simulink model "PlanarModelandControl"  (R2026a)
settings: Solver=FixedStepDiscrete  StopTime=StopTime  FixedStep=Ts  MaxStep=0  RelTol=1e-5  AbsTol=1e-3
PostLoadFcn:
  | Controles_Ativos = 1;
  | Vx_Ref = 0;
  | Vx_init = 0;
  | t_default = [0; 10000];
  | Brake_Cmd = [t_default, [0; 0]];
  | Accel_Cmd = [t_default, [0; 0]];
  | Steer_Cmd = [t_default, [0; 0]];
  | Flag_CruiseControl = 0;
  | Ts = 0.001;
  | load('TC_LUT.mat')
InitFcn:
  | % Carrega parâmetros gerais (Massa, Ganhos PID, Geometria)
  | run('Parameters.m');
  | 
  | % Carrega as Tabelas (Lookup Tables)
  | run('curva_steering.m');
  | run('curva_tq_motor.m');
  | load('TC_LUT.mat')
model workspace (MAT data in the model file):
  A = 1.22
  Ack = 1
  Cd = 1.1
  Cl = -2
  Cs = [19075.58 25992.8 31792.64 35898.02 39044.37]
  Fz0x = -811.9083
  Fz0y = -210.9467
  Fz_cs = [222.41 444.82 667.23 889.64 1112.05]
  IAY = 0
  IAx = 0
  IAy = 0
  Im = 0.00033
  It = 0.0094
  Iw = 0.1578
  Iw_ = 0.7
  Iz_car = 180
  K_50_understeer = 0.005184
  K_Stability = 1
  K_oversteer = -0.001
  Kappa_F = 0
  Kappa_R = 0
  Km_FM = 110000
  Km_Fm = 110000
  Km_RM = 120000
  Km_Rm = 120000
  KphiARB_F = 0
  KphiARB_R = 11000
  KphiF = 17054.5
  KphiR = 40034.8
  Kphim_F = 137500
  Kphim_R = 48600
  L = 1.53
  M = 300
  Mgt = 11.72
  P = 68.9476
  P0 = 55.4852
  R = 0.2032
  Rcurva = 20
  Re = 0.2
  SR = 3
  TV_On = 1
  Ts = 0.001
  W = 2940
  Wf = 308.034
  Wr = 339.426
  Ws = 2099.34
  YLL = 1.11022302463e-16
  cx1 = 1.3668
  cy1 = 1.839
  dpi = 0.242630467224
  dx1 = 2.1568
  dx2 = -0.065814
  dx3 = 0.0011254
  dy1 = 2.453
  dy2 = -0.11197
  dy3 = 10.2899
  ex1 = -0.19815
  ex2 = -0.62529
  ex3 = 1.3396
  ex4 = 0.0029464
  ey1 = 0.96085
  ey2 = -0.28662
  ey3 = 0.14389
  ey4 = 4.9274
  ey5 = -70.7165
  g = 9.81
  hcg = 0.252
  hcgs = 0.202
  hrc_f = 0.056
  hrc_r = 0.084
  hs_f = 0.222
  hs_r = 0.222
  hx1 = -0.003859
  hx2 = -0.000374
  hy1 = -0.000403
  hy2 = -0.000261
  kx1 = -36.9039
  kx2 = 22.3857
  kx3 = 0.61844
  ky1 = -83.2526
  ky2 = -5.565
  ky3 = 0.88491
  ky4 = 2.9994
  ky5 = -99.9393
  ky6 = -3.4705
  ky7 = -0.91452
  lf = 0.8415
  lr = 0.6885
  mass_FL = 67.5
  mass_FR = 67.5
  mass_RL = 82.5
  mass_RR = 82.5
  mi = 2
  ms = 240
  pty1 = 0.005744
  pty2 = 19.1334
  px1 = -0.039964
  px2 = -0.15015
  px3 = -0.32528
  px4 = -0.063273
  py1 = 0.38578
  py2 = 1.2048
  py3 = -0.14544
  py4 = 0.092886
  py5 = -0.82901
  r = 9
  rhoar = 1.22
  s_f = 1
  s_r = 0.9
  tf = 1.25
  tr = 1.25
  vx1 = -0.11922
  vx2 = 0.002205
  vy1 = 0.032907
  vy2 = 0.018068
  vy3 = -1.0429
  vy4 = 0.53712
  x_fl = 0.8415
  x_fr = 0.8415
  x_rl = 0.6885
  x_rr = 0.6885
  xs_f = 0.826
  xs_r = 0.704
  y_fl = 0.6
  y_fr = 0.6
  y_rl = 0.59
  y_rr = 0.59
values from neighbouring files (symbols this model uses):
  GG_mode = 0    [autocross.m]    (also in teste.mat)
  Gr = 11.72    [Parameters.m]    (also in teste.mat)
  K_variavel = [-0.001, 0, 0.0008, 0.0012, 0.0015]    [Parameters.m]    (also in teste.mat = [-0.001 0 0.0008 0.0012 0.0015])
  Ki_data = [283117.9493, 114831.615, 51596.0519, 30556.5344, 17525.221, 11773.8301, 10567.5001]*0.008    [Parameters.m]    (also in teste.mat = [2264.9435944 918.65292 412.7684152 244.4522752 140.201768 9)
  Kp_data = [2877.5702, 2303.9999, 1972.0835, 1556.8775, 1258.2451, 1022.7085, 982.7861, ]*5    [Parameters.m]    (also in teste.mat = [14387.851 11519.9995 9860.4175 7784.3875 6291.2255 5113.542)
  Mz_maxF = [0 66.3 132.6 198.9 265.2 330.23 396.52 462.83 529.112 595.42 661.73 728.03 794.33 860.62 926.92 993.23 1058.25 1124.55 1190.85 1257.15 1323.45 1389.75 1456.05 1522.35 1588.65 1654.95]    [Parameters.m]    (also in teste.mat = [0 66.3 132.6 198.9 265.2 330.23 396.52 462.83 529.112 595.4)
  Mz_minF = [0 -66.3 -132.6 -198.9 -265.2 -330.23 -396.52 -462.83 -529.112 -595.42 -661.73 -728.03 -794.33 -860.62 -926.92 -993.23 -1058.25 -1124.55 -1190.85 -1257.15 -1323.45 -1389.75 -1456.05 -1522.35 -1588.65 -1654.95]    [Parameters.m]    (also in teste.mat = [0 -66.3 -132.6 -198.9 -265.2 -330.23 -396.52 -462.83 -529.1)
  pedal = [0 4 8 12 16 20 24 28 32 36 40 44 48 52 56 60 64 68 72 76 80 84 88 92 96 100]    [Parameters.m]    (also in teste.mat = [0 4 8 12 16 20 24 28 32 36 40 44 ... (26 elements, 1x26)])
  rpm_355_novo = [0 10050 10100 10140 10170 10210 10250 10280 10320 10360         10390 10420 10460 10620 10770 10920 11050 11170 11280         11380 11470 11550 11625 11680 11730 11775 11790 11800]    [curva_tq_motor.m]    (also in teste.mat = [0 10050 10100 10140 10170 10210 10250 10280 10320 10360 103)
  Steering_inner = [-29.018 ... (first row only)    [curva_steering.m]    (also in teste.mat = [-29.018 -25.595 -22.357 -19.265 -16.292 -13.415 -10.619 -7.)
  Steering_outer = [-25.111 ... (first row only)    [curva_steering.m]    (also in teste.mat = [-25.111 -22.589 -20.082 -17.585 -15.094 -12.604 -10.11 -7.6)
  steering_vol = [-105 ... (first row only)    [curva_steering.m]    (also in teste.mat = [-105 -94.5 -84 -73.5 -63 -52.5 -42 -31.5 -21 -10.5 0 10.5 .)
  torque_355_novo = [16.2 16.2 16.0 15.8 15.6 15.4 15.2 15.0 14.8 14.6            14.4 14.2 14.0 13.0 12.0 11.0 10.0 9.0 8.0            7.0 6.0 5.0 4.0 3.0 2.0 1.0 0.5 0.0]    [curva_tq_motor.m]    (also in teste.mat = [16.2 16.2 16 15.8 15.6 15.4 15.2 15 14.8 14.6 14.4 14.2 ...)
  Vx = [0, 12.5, 23.6, 30, 35]    [Parameters.m]    (also in teste.mat = [0 12.5 23.6 30 35])
  Vx_axis = [5, 10, 15, 20, 25, 30, 35]    [Parameters.m]    (also in teste.mat = [5 10 15 20 25 30 35])
undefined symbols (not defined in the file or in its folder): DiscIntNeverNeededParam, magnitude
libraries: simulink x5, fuzblock x4, sl_discontinuities x3, simulink_extras x3, slpidlib x1
(part 1 of 4)
settings Model: SimulationMode=accelerator  SILPILModeSetting=simonly  SILPILSimulationModeTopModel=accelerator  PauseTimes=21.560  Creator=lucas  LastModifiedBy=lucaspz
settings SolverCC: ZeroCrossAlgorithm=Adaptive
settings DebuggingCC: SignalInfNanChecking=warning  IgnoredZcDiagnostic=none
settings SimscapeCC: SimscapeLogDecimation=1  SimscapeLogDataHistory=5000  SimscapeLogType=none  SimscapeCacheCompileArtifacts=�  SimscapeAutoSelectReusableComponents=�  SimscapeNominalValues="[{\"value\":\"1\",\"unit\":\"A\"},{\"value\":\"1\",\"unit\":\"bar\"},{\"value\":\"1\",\"unit\":\"cm^2\"},{\"value\":\"1\",\"unit\":\"cm^3/s\"},{\"value\":\"1\",\"unit\":\"kJ/kg\"},{\"value\":\"1\",\"unit\":\"kW\"},{\"value\":\"1\",\"unit\":\"l\"},{\"value\":\"1\",\"unit\":\"N\"},{\"value\":\"1\",\"unit\":\"N*m\"},{\"value\":\"1\",\"unit\":\"V\"}]"

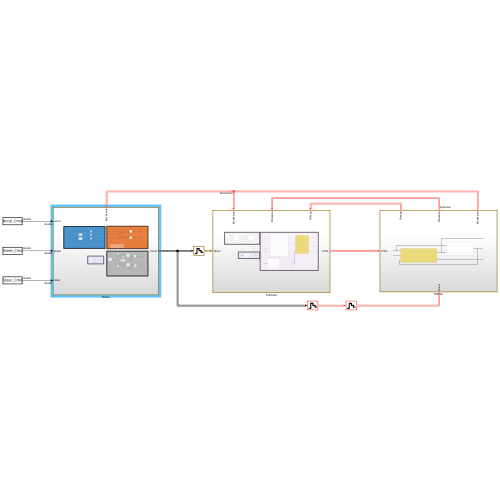
[thumbnail: Simulink's stored preview of the top-level diagram]
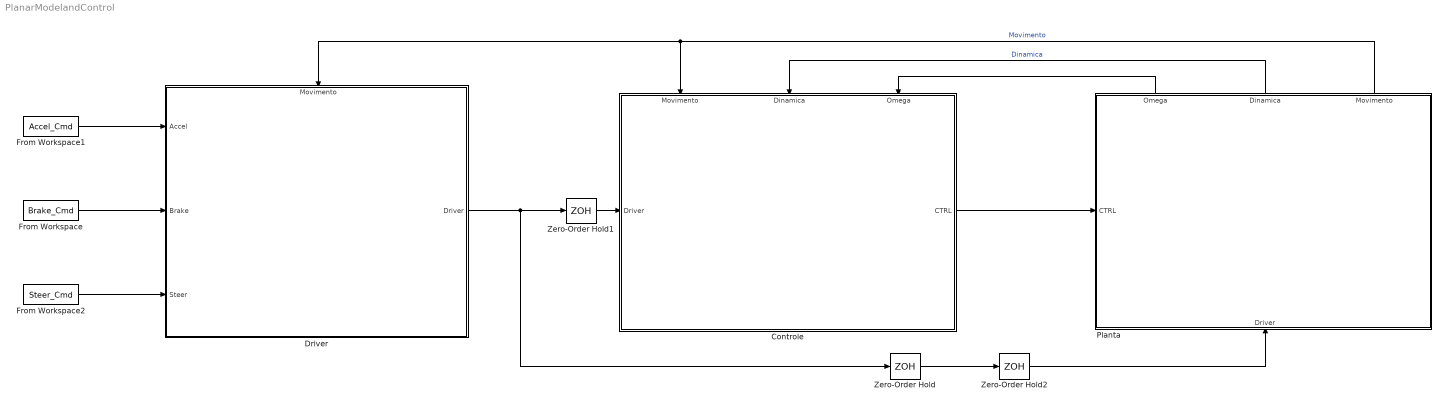
== system PlanarModelandControl ==
  Controle : Subsystem
      settings: PortSchema="{\"entries\":[{\"content\":{\"sides\":[{\"content\":{\"connectorIds\":[\"In1\"]},\"type\":\"ConnectorPlacement.EquallySpacedRectSide\",\"uuid\":\"56151e0a-bfa8-4f54-a24f-2d0820c0f72f\"},{\"content\":{\"connectorIds\":[\"Out1\"],\"side\":\"RIGHT\"},\"type\":\"ConnectorPlacement.EquallySpacedRectSide\",\"uuid\":\"009f5130-1eb0-405e-8bd9-87ce9896eab4\"},{\"content\":{\"connectorIds\":[\"In2\",\"In3\",\"In4\"],\"side\":\"TOP\"},\"type\":\"ConnectorPlacement.EquallySpacedRectSide\",\"uuid\":\"a6bcbcd6-40f0-4fd8-bd63-4ee066739afa\"},{\"content\":{\"side\":\"BOTTOM\"},\"type\":\"ConnectorPlacement.EquallySpacedRectSide\",\"uuid\":\"b3b13c4c-f99f-4d3e-8267-66104372a3a2\"}]},\"type\":\"ConnectorPlacement.EquallySpacedConnector\",\"uuid\":\"073272d2-a218-475e-9766-74f02117ed8f\"}],\"packageUris\":[\"http://schema.mathworks.com/mf0/Connector/R2020b\"],\"version\":\"1.0\"}"
  Driver : Subsystem
      settings: PortSchema="{\"entries\":[{\"content\":{\"sides\":[{\"content\":{\"connectorIds\":[\"In1\",\"In2\",\"In3\"]},\"type\":\"ConnectorPlacement.EquallySpacedRectSide\",\"uuid\":\"443768bc-c7a3-4466-aec1-096d5f7be67d\"},{\"content\":{\"connectorIds\":[\"Out1\"],\"side\":\"RIGHT\"},\"type\":\"ConnectorPlacement.EquallySpacedRectSide\",\"uuid\":\"da7fa586-0510-4892-a092-db6f1a27a2ae\"},{\"content\":{\"connectorIds\":[\"In4\"],\"side\":\"TOP\"},\"type\":\"ConnectorPlacement.EquallySpacedRectSide\",\"uuid\":\"2c1d5f3e-1036-4d42-9b19-272a34ee3261\"}]},\"type\":\"ConnectorPlacement.EquallySpacedConnector\",\"uuid\":\"e3719b6f-9fe7-4b22-84b4-1211ae849bc8\"}],\"packageUris\":[\"http://schema.mathworks.com/mf0/Connector/R2020b\"],\"version\":\"1.0\"}"
  From Workspace : FromWorkspace  VariableName=Brake_Cmd
  From Workspace1 : FromWorkspace  VariableName=Accel_Cmd
  From Workspace2 : FromWorkspace  VariableName=Steer_Cmd
  Planta : Subsystem
      settings: PortSchema="{\"entries\":[{\"content\":{\"sides\":[{\"content\":{\"connectorIds\":[\"In1\"]},\"type\":\"ConnectorPlacement.EquallySpacedRectSide\",\"uuid\":\"52b90d9a-1301-422a-8be6-7849c42227ef\"},{\"content\":{\"side\":\"RIGHT\"},\"type\":\"ConnectorPlacement.EquallySpacedRectSide\",\"uuid\":\"dc52c6c3-a926-4968-a1e6-05499ba453a3\"},{\"content\":{\"connectorIds\":[\"Out1\",\"Out2\",\"Out3\"],\"side\":\"TOP\"},\"type\":\"ConnectorPlacement.EquallySpacedRectSide\",\"uuid\":\"4b5251a8-57d3-4280-b8f2-cf87664bd53d\"},{\"content\":{\"connectorIds\":[\"In2\"],\"side\":\"BOTTOM\"},\"type\":\"ConnectorPlacement.EquallySpacedRectSide\",\"uuid\":\"b553b3b1-29bc-46a4-a447-2da3a1af9f5a\"}]},\"type\":\"ConnectorPlacement.EquallySpacedConnector\",\"uuid\":\"f03778a7-5380-42c5-afb8-9cbdce520324\"}],\"packageUris\":[\"http://schema.mathworks.com/mf0/Connector/R2020b\"],\"version\":\"1.0\"}"
  Zero-Order Hold : ZeroOrderHold  SampleTime=0.001
  Zero-Order Hold1 : ZeroOrderHold  SampleTime=0.01
  Zero-Order Hold2 : ZeroOrderHold  SampleTime=0.001
  wires:
  Driver/1(Driver) -> Zero-Order Hold/1, Zero-Order Hold1/1
  Zero-Order Hold/1 -> Zero-Order Hold2/1
  Controle/1(CTRL) -> Planta/1(CTRL)
  Planta/2(Dinamica) -> Controle/3(Dinamica)  "Dinamica"
  Planta/3(Movimento) -> Driver/4(Movimento), Controle/2(Movimento)  "Movimento"
  Planta/1(Omega) -> Controle/4(Omega)
  From Workspace1/1 -> Driver/1(Accel)
  From Workspace/1 -> Driver/2(Brake)
  From Workspace2/1 -> Driver/3(Steer)
  Zero-Order Hold1/1 -> Controle/1(Driver)
  Zero-Order Hold2/1 -> Planta/2(Driver)

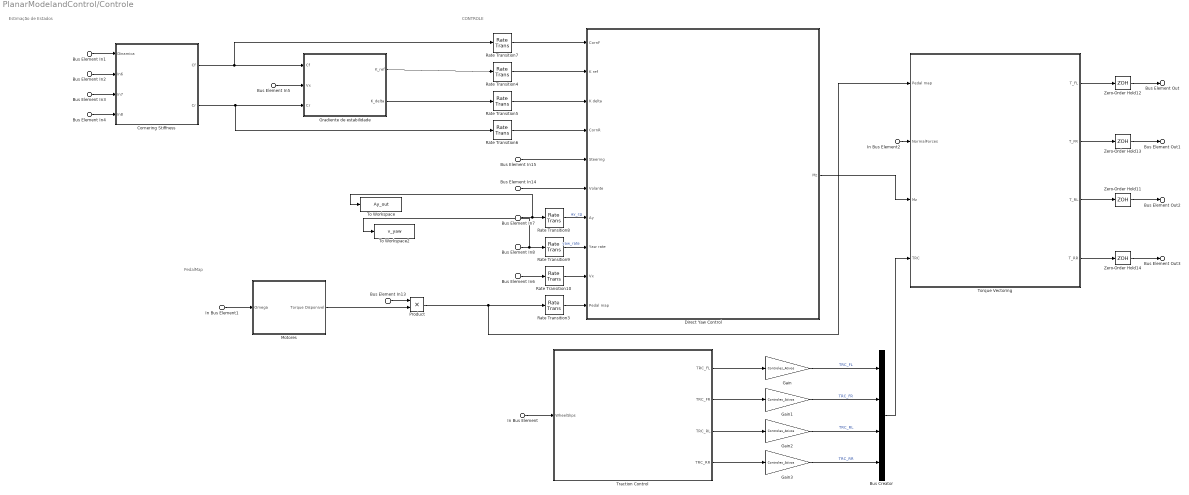
== system PlanarModelandControl/Controle ==
  note: PedalMap
  note: CONTROLE
  note: Estimação de Estados
  Bus Element In13 : Inport  PortName=Driver  Element=AccCmd
  Bus Element In14 : Inport  PortName=Driver  Element=volante
  Bus Element In15 : Inport  PortName=Driver  Element=steering_inner
  Bus Element In5 : Inport  Port=2  PortName=Movimento  Element=Vx_cg
  Bus Element In6 : Inport  Port=2  PortName=Movimento  Element=Vx_cg
  Bus Element In7 : Inport  Port=2  PortName=Movimento  Element=Ay_cg
  Bus Element In8 : Inport  Port=2  PortName=Movimento  Element=Yaw.v_yaw
  In Bus Element2 : Inport  Port=3  PortName=Dinamica  Element=NormalForces
  Bus Element In1 : Inport  Port=3  PortName=Dinamica  Element=NormalForces.Fz_FL
  Bus Element In2 : Inport  Port=3  PortName=Dinamica  Element=NormalForces.Fz_FR
  Bus Element In3 : Inport  Port=3  PortName=Dinamica  Element=NormalForces.Fz_RL
  Bus Element In4 : Inport  Port=3  PortName=Dinamica  Element=NormalForces.Fz_RR
  In Bus Element : Inport  Port=3  PortName=Dinamica  Element=WheelSlips
  In Bus Element1 : Inport  Port=4  PortName=Omega
  Bus Creator : BusCreator  Inputs=4
  Cornering Stiffness : Subsystem
  Direct Yaw Control : Subsystem
  Gain{,1..3} (4 alike) : Gain  Gain=Controles_Ativos
  Gradiente de estabilidade : Subsystem
  Motores : Subsystem
  Product : Product
  Rate Transition{3..10} (8 alike) : RateTransition  OutPortSampleTime=0.01
  To Workspace : ToWorkspace  VariableName=Ay_out  SaveFormat=Structure With Time  SampleTime=-1
  To Workspace2 : ToWorkspace  VariableName=v_yaw  SaveFormat=Structure With Time  SampleTime=-1
  Torque Vectoring : Subsystem  TreatAsAtomicUnit=on
  Traction Control : Subsystem
  Zero-Order Hold{11..14} (4 alike) : ZeroOrderHold  SampleTime=0.001
  Bus Element Out : Outport  VectorParamsAs1DForOutWhenUnconnected=off  PortName=CTRL  Element=T_FL
  Bus Element Out1 : Outport  VectorParamsAs1DForOutWhenUnconnected=off  PortName=CTRL  Element=T_FR
  Bus Element Out2 : Outport  VectorParamsAs1DForOutWhenUnconnected=off  PortName=CTRL  Element=T_RL
  Bus Element Out3 : Outport  VectorParamsAs1DForOutWhenUnconnected=off  PortName=CTRL  Element=T_RR
  wires:
  Bus Element In13/1 -> Product/1
  Bus Element In3/1 -> Cornering Stiffness/3(In7)
  Bus Element In1/1 -> Cornering Stiffness/1(Dinamica)
  Bus Element In4/1 -> Cornering Stiffness/4(In8)
  Bus Element In2/1 -> Cornering Stiffness/2(In6)
  Bus Element In14/1 -> Direct Yaw Control/6(Volante)
  Bus Element In7/1 -> To Workspace/1, Rate Transition8/1
  Bus Element In6/1 -> Rate Transition10/1
  Bus Element In15/1 -> Direct Yaw Control/5(Steering)
  Cornering Stiffness/1(Cf) -> Rate Transition7/1, Gradiente de estabilidade/1(Cf)
  Gradiente de estabilidade/2(K_delta) -> Rate Transition5/1
  Gradiente de estabilidade/1(K_ref) -> Rate Transition4/1
  Cornering Stiffness/2(Cr) -> Gradiente de estabilidade/3(Cr), Rate Transition6/1
  Torque Vectoring/1(T_FL) -> Zero-Order Hold12/1
  Torque Vectoring/2(T_FR) -> Zero-Order Hold13/1
  Torque Vectoring/4(T_RR) -> Zero-Order Hold14/1
  Bus Element In5/1 -> Gradiente de estabilidade/2(Vx)
  Motores/1(Torque Disponivel) -> Product/2
  Zero-Order Hold13/1 -> Bus Element Out1/1
  Zero-Order Hold11/1 -> Bus Element Out2/1
  Zero-Order Hold14/1 -> Bus Element Out3/1
  Gain/1 -> Bus Creator/1  "TRC_FL"
  Gain1/1 -> Bus Creator/2  "TRC_FR"
  Rate Transition3/1 -> Direct Yaw Control/10(Pedal map)
  Rate Transition4/1 -> Direct Yaw Control/2(K ref)
  Rate Transition5/1 -> Direct Yaw Control/3(K delta)
  Rate Transition6/1 -> Direct Yaw Control/4(CornR)
  Rate Transition7/1 -> Direct Yaw Control/1(CornF)
  Rate Transition8/1 -> Direct Yaw Control/7(Ay)  "ay_cg"
  Rate Transition9/1 -> Direct Yaw Control/8(Yaw rate)  "yaw_rate"
  Rate Transition10/1 -> Direct Yaw Control/9(Vx)
  Gain2/1 -> Bus Creator/3  "TRC_RL"
  Gain3/1 -> Bus Creator/4  "TRC_RR"
  Bus Element In8/1 -> To Workspace2/1, Rate Transition9/1
  Torque Vectoring/3(T_RL) -> Zero-Order Hold11/1
  Zero-Order Hold12/1 -> Bus Element Out/1
  Direct Yaw Control/1(Mz) -> Torque Vectoring/3(Mz)
  Traction Control/1(TRC_FL) -> Gain/1
  Traction Control/2(TRC_FR) -> Gain1/1
  Traction Control/3(TRC_RL) -> Gain2/1
  Traction Control/4(TRC_RR) -> Gain3/1
  Bus Creator/1 -> Torque Vectoring/4(TRC)
  In Bus Element/1 -> Traction Control/1(WheelSlips)
  In Bus Element1/1 -> Motores/1(Omega)
  In Bus Element2/1 -> Torque Vectoring/2(NormalForces)
  Product/1 -> Torque Vectoring/1(Pedal map), Rate Transition3/1

== system PlanarModelandControl/Driver ==
  note: positivo para esquerda --> left = inner e right = outer
  note: Curva de velocidade
  note: Indicador Brake/Acc
  note: Curva de Steering
  note: Indicador inner/outer
  note: negativo para direita --> left = outer e right = inner
  Bus Element In1 : Inport  PortName=Accel
  Bus Element In2 : Inport  Port=2  PortName=Brake
  Bus Element In3 : Inport  Port=3  PortName=Steer
  Bus Element In : Inport  Port=4  PortName=Movimento  Element=Vx_cg
  1-D Lookup Table2 : Lookup_n-D  NumberOfTableDimensions=1  BreakpointsForDimension1=steering_vol  Table=Steering_inner  InternalRulePriority=Speed  RndMeth=Simplest
  1-D Lookup Table3 : Lookup_n-D  NumberOfTableDimensions=1  BreakpointsForDimension1=steering_vol  Table=Steering_outer  InternalRulePriority=Speed  RndMeth=Simplest
  Abs : Abs  SaturateOnIntegerOverflow=off
  Constant : Constant  Value=0
  Constant1 : Constant  Value=0
  Constant12 : Constant  Value=Flag_CruiseControl
  Constant2 : Constant  Value=Vx_Ref
  Degrees to Radians : simulink_extras/Transformations/Degrees to Radians
  Degrees to Radians1 : simulink_extras/Transformations/Degrees to Radians
  Degrees to Radians2 : simulink_extras/Transformations/Degrees to Radians
  PID Controller : slpidlib/PID Controller  Controller=PI  Form=Parallel  TimeDomain=Discrete-time  UseExternalTs=off  SampleTime=0.0100000000000000002  IntegratorMethod=Forward Euler  FilterMethod=Forward Euler  ControllerParametersSource=internal  P=0.500000000000000  I=0.010000000000000  UseKiTs=off  D=0.004962206866398  UseExternalDerivativeSource=off  N=0.000683414490221  UseFilter=on  TunerSelectOption=Frequency Response Based  InitialConditionSource=internal  InitialConditionForIntegrator=0  InitialConditionForFilter=0  DifferentiatorICPrevScaledInput=0  ExternalReset=none  TrackingMode=off  Kt=1  LimitOutput=on  SatLimitsSource=internal  UpperSaturationLimit=1  LowerSaturationLimit=-1  LinearizeAsGain=on  AntiWindupMode=none  Kb=1  LimitIntegrator=off  UpperIntegratorSaturationLimit=inf  LowerIntegratorSaturationLimit=-inf  POutMin=[]  POutMax=[]  IOutMin=[]  IOutMax=[]  DOutMin=[]  DOutMax=[]  NOutMin=[]  NOutMax=[]  SumOutMin=[]  SumOutMax=[]  SaturationOutMin=[]  SaturationOutMax=[]  PParamMin=[]  PParamMax=[]  IParamMin=[]  IParamMax=[]  DParamMin=[]  DParamMax=[]  NParamMin=[]  NParamMax=[]  KbParamMin=[]  KbParamMax=[]  KtParamMin=[]  KtParamMax=[]  KbOutMin=[]  KbOutMax=[]  KtOutMin=[]  KtOutMax=[]  IntegratorOutMin=[]  IntegratorOutMax=[]  FilterOutMin=[]  FilterOutMax=[]  SumI1OutMin=[]  SumI1OutMax=[]  SumI2OutMin=[]  SumI2OutMax=[]  SumI3OutMin=[]  SumI3OutMax=[]  SumI4OutMin=[]  SumI4OutMax=[]  SumDOutMin=[]  SumDOutMax=[]  DifferentiatorOutMin=[]  DifferentiatorOutMax=[]  FilterDiffOutCoefMin=[]  FilterDiffOutCoefMax=[]  ReciprocalOutMin=[]  ReciprocalOutMax=[]  SumDenOutMin=[]  SumDenOutMax=[]  SumNumOutMin=[]  SumNumOutMax=[]  DivideOutMin=[]  DivideOutMax=[]  UdiffTsProdOutMin=[]  UdiffTsProdOutMax=[]  NTsProdOutMin=[]  NTsProdOutMax=[]  UintegralTsProdOutMin=[]  UintegralTsProdOutMax=[]  UngainTsProdOutMin=[]  UngainTsProdOutMax=[]  ClampingZeroOutDataTypeStr=Inherit: Inherit via back propagation  ClampingZeroOutMin=[]  ClampingZeroOutMax=[]  FilterDenConstantOutDataTypeStr=Inherit: Inherit via back propagation  FilterDenConstantOutMin=[]  FilterDenConstantOutMax=[]  PCopyOutDataTypeStr=Inherit: Inherit via back propagation  PCopyOutMin=[]  PCopyOutMax=[]  NCopyOutDataTypeStr=Inherit: Inherit via back propagation  NCopyOutMin=[]  NCopyOutMax=[]  IntegratorContinuousStateAttributes=''  IntegratorStateMustResolveToSignalObject=off  IntegratorRTWStateStorageClass=Auto  FilterContinuousStateAttributes=''  FilterStateMustResolveToSignalObject=off  FilterRTWStateStorageClass=Auto  ParallelPVariant=InternalParameters  IdealPVariant=Disabled  IVariant=InternalParameters  DVariant=Disabled  IntegratorVariant=Discrete  SatVariant=Enabled  AWVariant=Passthrough  PCopyVariant=Disabled  TRVariant=Disabled  FdbkBlocksVariant=Forward  IdealPFdbkVariant=Disabled  SatFdbkVariant=Disabled  DerivativeFilterVariant=Disabled  NVariant=Disabled  NCopyVariant=Disabled_SignalSpec  FilterICVariant=Disabled  IntegratorICVariant=InternalICs  ExternalResetVariant=Disabled  TRSumVariant=Passthrough  SumFdbkVariant=Disabled  SumVariant=Sum_PI  TsampFilterVariant=InternalTs  TsampNgainVariant=Passthrough  TsampIntegralVariant=Disabled_SignalSpec  ExternalSaturationOutDataTypeStr=Inherit: Same as second input  DerivativeSourceVariant=Disabled  PreIntVariant=InternalPreInt
  Saturation : Saturate  UpperLimit=1000  LowerLimit=0
  Sum : Sum  Inputs=|+-
  Switch : Switch  SaturateOnIntegerOverflow=off  InputSameDT=off
  Switch1 : Switch  SaturateOnIntegerOverflow=off  InputSameDT=off
  Switch{2..3,5..6} (4 alike) : Switch  Criteria=u2 > Threshold  SaturateOnIntegerOverflow=off  InputSameDT=off
  Bus Element Out : Outport  PortName=Driver  Element=BrakeCmd
  Bus Element Out1 : Outport  PortName=Driver  Element=AccCmd
  Bus Element Out4 : Outport  PortName=Driver  Element=steering_inner
  Bus Element Out5 : Outport  PortName=Driver  Element=steering_outer
  Bus Element Out2 : Outport  PortName=Driver  Element=volante
  wires:
  Constant/1 -> Switch1/1
  Abs/1 -> Switch1/3
  Bus Element In/1 -> Sum/2  "vel_atual"
  Constant1/1 -> Switch/3
  Switch3/1 -> Bus Element Out5/1  "outer"
  Switch2/1 -> Bus Element Out4/1  "inner"
  Degrees to Radians/1 -> Bus Element Out2/1, Switch3/2, Switch2/2
  Degrees to Radians2/1 -> Switch3/1, Switch2/3
  Degrees to Radians1/1 -> Switch2/1, Switch3/3
  1-D Lookup Table2/1 -> Degrees to Radians1/1  "steering_volante"
  1-D Lookup Table3/1 -> Degrees to Radians2/1  "steering_volante"
  PID Controller/1 -> Switch/2, Switch/1, Abs/1, Switch1/2  "PID"
  Sum/1 -> PID Controller/1
  Saturation/1 -> Sum/1
  Constant2/1 -> Saturation/1
  Switch5/1 -> Bus Element Out/1
  Constant12/1 -> Switch6/2, Switch5/2
  Switch/1 -> Switch6/1
  Switch1/1 -> Switch5/1
  Bus Element In1/1 -> Switch6/3
  Bus Element In2/1 -> Switch5/3
  Switch6/1 -> Bus Element Out1/1
  Bus Element In3/1 -> Degrees to Radians/1, 1-D Lookup Table3/1, 1-D Lookup Table2/1  "Steer"

== system PlanarModelandControl/Planta ==
  CTRL : Inport
  Driver : Inport  Port=2
  Dinamica do Veículo : Subsystem
  Movimento do Veiculo : Subsystem
  Omega : Outport
  Dinamica : Outport  Port=2
  Movimento : Outport  Port=3
  wires:
  CTRL/1 -> Dinamica do Veículo/2(CTRL)
  Dinamica do Veículo/1(Dinamica) -> Dinamica/1, Movimento do Veiculo/2(Dinamica)
  Movimento do Veiculo/1(Movimento) -> Dinamica do Veículo/3(Movimento), Movimento/1
  Dinamica do Veículo/2(Omega) -> Omega/1
  Driver/1 -> Movimento do Veiculo/1(Driver), Dinamica do Veículo/1(Driver)

== system PlanarModelandControl/Controle/Cornering Stiffness ==
  Dinamica : Inport
  In6 : Inport  Port=2
  In7 : Inport  Port=3
  In8 : Inport  Port=4
  1-D Lookup Table{,1..3} (4 alike) : Lookup_n-D  NumberOfTableDimensions=1  BreakpointsForDimension1=Fz_cs  Table=Cs  InternalRulePriority=Speed  RndMeth=Simplest
  Sum : Sum  Inputs=|++
  Sum1 : Sum  Inputs=|++
  Cf : Outport
  Cr : Outport  Port=2
  wires:
  1-D Lookup Table1/1 -> Sum1/2
  In7/1 -> 1-D Lookup Table2/1
  Sum/1 -> Cr/1
  Sum1/1 -> Cf/1
  Dinamica/1 -> 1-D Lookup Table/1
  In6/1 -> 1-D Lookup Table1/1
  1-D Lookup Table3/1 -> Sum/2
  1-D Lookup Table/1 -> Sum1/1
  1-D Lookup Table2/1 -> Sum/1
  In8/1 -> 1-D Lookup Table3/1

== system PlanarModelandControl/Controle/Direct Yaw Control ==
  CornF : Inport
  K ref : Inport  Port=2
  K delta : Inport  Port=3
  CornR : Inport  Port=4
  Steering : Inport  Port=5
  Volante : Inport  Port=6
  Ay : Inport  Port=7
  Yaw rate : Inport  Port=8
  Vx : Inport  Port=9
  Pedal map : Inport  Port=10
  Constant : Constant  Value=TV_On
  Gain : Gain  Gain=Controles_Ativos
  MDYC : Subsystem
  ON/OFF : Subsystem
  Rate Transition : RateTransition  OutPortSampleTime=0.001
  To Workspace1 : ToWorkspace  VariableName=Yaw_des  SaveFormat=Structure With Time  SampleTime=-1
  Yaw desejado : Subsystem
  Mz : Outport
  wires:
  Steering/1 -> Yaw desejado/1(Steering)
  K ref/1 -> Yaw desejado/2(K ref)
  Vx/1 -> MDYC/2(Vx), ON/OFF/4(Vx), Yaw desejado/3(Vx)
  Yaw desejado/1(Yaw_des) -> Rate Transition/1, MDYC/1(Yaw_des)  "yaw_ref"
  Yaw rate/1 -> MDYC/3(Yaw rate)
  Pedal map/1 -> MDYC/4(Pedal map)
  CornR/1 -> MDYC/5(CornR)
  CornF/1 -> MDYC/6(CornF)
  K delta/1 -> MDYC/7(K delta)
  Ay/1 -> MDYC/8(Ay)
  Volante/1 -> ON/OFF/1(Volante)
  Constant/1 -> ON/OFF/2(TV_On)
  MDYC/1(Mz_ajustado) -> ON/OFF/3(Mz_ajustado)
  ON/OFF/1(Mz) -> Gain/1
  Gain/1 -> Mz/1
  Rate Transition/1 -> To Workspace1/1
